simulink model "v01_aa_rii_16r4t11f_ver121sa"  (Simulink 7.6)
settings: Solver=ode45  StartTime=0.0  StopTime=128000  RelTol=1e-3
undefined symbols (not defined in the file or in its folder): sfp
libraries: xbsIndex_r4 x27287, monroe_library x154, xbsReplacements_r4 x78, casper_library_misc x33, simulink x32, dspsigops x32, xps_library x24
(part 24 of 178)

== system v01_aa_rii_16r4t11f_ver121sa/pfb_fir_real2/coeff_gen_6_9 ==
  sync : Inport
  Counter0 : xbsIndex_r4/Counter  cnt_type=Free Running  cnt_to=Inf  operation=Up  start_count=8  cnt_by_val=1  arith_type=Unsigned  n_bits=7  bin_pt=0  load_pin=off  rst=on  en=off  explicit_period=on  period=1  dbl_ovrd=off  use_behavioral_HDL=off  implementation=Fabric  block_type=counter
  Counter1 : xbsIndex_r4/Counter  cnt_type=Free Running  cnt_to=Inf  operation=Down  start_count=119  cnt_by_val=1  arith_type=Unsigned  n_bits=7  bin_pt=0  load_pin=off  rst=on  en=off  explicit_period=on  period=1  dbl_ovrd=off  use_behavioral_HDL=off  implementation=Fabric  block_type=counter
  Delay31 : xbsIndex_r4/Delay  rst=off  infoeditControl=Selection of Reset will increase slice count due to use of real FFs and instead of SRLs  en=off  latency=2  dbl_ovrd=off  reg_retiming=off  block_type=delay
  Delay32 : xbsIndex_r4/Delay  rst=off  infoeditControl=Selection of Reset will increase slice count due to use of real FFs and instead of SRLs  en=off  latency=1  dbl_ovrd=off  reg_retiming=off  block_type=delay
  Delay33 : xbsIndex_r4/Delay  rst=off  infoeditControl=Selection of Reset will increase slice count due to use of real FFs and instead of SRLs  en=off  latency=1  dbl_ovrd=off  reg_retiming=off  block_type=delay
  Delay34 : xbsIndex_r4/Delay  rst=off  infoeditControl=Selection of Reset will increase slice count due to use of real FFs and instead of SRLs  en=off  latency=2  dbl_ovrd=off  reg_retiming=off  block_type=delay
  coeff_ram_1 : xbsIndex_r4/Dual Port RAM  depth=128  initVector=[-0.000127160545753068 -0.000442154443954354 -0.000760500021931522 -0.00108286593169467 -0.00140992727613395 -0.00174236399262348 -0.00208085920782171 -0.00242609756580559 -0.00277876353172613 -0.0031395396732198 -0.00350910492185494 -0.00388813281693438 -0.00427728973401434 -0.00467723310053645 -0.00508860960100282 -0.00551205337415503 -0.00594818420464534 -0.00639760571171294 -0.00686090353739995 -0.00733864353685997 -0.00783136997332745 -0.00833960372032831 -0.00886384047372118 -0.00940454897616429 -0.00996216925660562 -0.0105371108873929 -0.0111297512615966 -0.0117404338931308 -0.012369466742247 -0.0130171205689619 -0.0136836273169615 -0.0143691785305073 -0.0150739238068405 -0.0157979692865596 -0.0165413761844115 -0.0173041593629059 -0.0180862859511236 -0.0188876740110524 -0.0197081912537385 -0.0205476538074967 -0.0214058250403737 -0.0222824144390066 -0.0231770765459626 -0.0240894099575895 -0.0250189563843456 -0.025965199775512 -0.0269275655101279 -0.0279054196559168 -0.0288980682979016 -0.0299047569383341 -0.0309246699694849 -0.0319569302207636 -0.0330005985815571 -0.0340546737010912 -0.0351180917665341 -0.0361897263604755 -0.0372683883988247 -0.0383528261500787 -0.0394417253368223 -0.0405337093202253 -0.0416273393682069 -0.042721115007841 -0.0438134744624772 -0.0449027951739552 -0.0459873944101843 -0.0470655299582668 -0.0481354009032336 -0.0491951484923634 -0.0502428570849517 -0.0512765551872898 -0.0522942165725149 -0.0532937614848831 -0.0542730579279157 -0.0552299230357647 -0.0561621245270369 -0.0570673822402172 -0.0579433697497217 -0.0587877160615143 -0.0595980073871155 -0.0603717889947299 -0.0611065671361221 -0.0617998110477661 -0.0624489550247014 -0.0630514005654251 -0.0636045185860608 -0.0641056517019449 -0.0645521165746833 -0.0649412063226375 -0.0652701929927136 -0.0655363300912374 -0.0657368551716176 -0.0658689924764135 -0.0659299556313471 -0.065916950388718 -0.0658271774176071 -0.065657835138181 -0.0654061225973403 -0.0650692423828866 -0.0646444035733198 -0.0641288247203163 -0.063519736860879 -0.0628143865560972 -0.0620100389534008 -0.0611039808691464 -0.0600935238883252 -0.0589760074781449 -0.0577488021121952 -0.0564093124018754 -0.0549549802317284 -0.0533832878953016 -0.0516917612281279 -0.0498779727344022 -0.0479395447039121 -0.0458741523157694 -0.0436795267254782 -0.0413534581318762 -0.0388937988204769 -0.0362984661797526 -0.0335654456868968 -0.0306927938596214 -0.0276786411705567 -0.0245211949208399 -0.021218742069504 -0.0177696520153009 -0.0141723793276274 -0.0104254664232543 -0.00652754618559848 -0.00247734452331941]  distributed_mem=Block RAM  init_a=0  init_b=0  rst_a=off  rst_b=off  en_a=off  en_b=off  latency=2  write_mode_A=No Read On Write  write_mode_B=No Read On Write  dbl_ovrd=off  optimize=Area  block_type=dpram
  coeff_ram_2 : xbsIndex_r4/Dual Port RAM  depth=128  initVector=[0.00172631713493355 0.00608451941274449 0.0105982426281969 0.0152683645091936 0.0200956579847837 0.0250807890553853 0.0302243147447241 0.0355266811362388 0.0409882214966272 0.0466091544891328 0.0523895824790868 0.0583294899341408 0.0644287419215347 0.0706870827046562 0.0771041344410556 0.0836793959839828 0.0904122417894169 0.0973019209304556 0.104347556220831 0.111548143449209 0.118902550725827 0.12640951794291 0.13406765635019 0.141875448246753 0.149831246790306 0.157933275924849 0.166179630427611 0.174568276076004 0.183097049935197 0.191763660766828 0.200565689559221 0.209500590179345 0.218565690146664 0.227758191528855 0.237075171959277 0.246513585775932 0.256070265281535 0.265741922124186 0.275525148798008 0.285416420262988 0.295412095683125 0.305508420281884 0.3157015273138 0.325987440150977 0.336362074483092 0.346821240629391 0.357360645961044 0.367975897432114 0.378662504217261 0.389415880454205 0.400231348088824 0.411104139820711 0.422029402146814 0.433002198500771 0.444017512485363 0.455070251195456 0.466155248628685 0.477267269181019 0.488401011224277 0.499551110762549 0.510712145164397 0.521878636967618 0.533045057753271 0.544205832085582 0.555355341514271 0.566487928635764 0.577597901209684 0.588679536326957 0.599727084625781 0.610734774551675 0.621696816657757 0.632607407941328 0.643460736212841 0.654250984493227 0.664972335435562 0.675618975766998 0.686185100746851 0.696664918636714 0.70705265517845 0.717342558075884 0.727528901476001 0.73760599044547 0.747568165438271 0.757409806750234 0.767125338956278 0.776709235326166 0.786156022214578 0.795460283421358 0.804616664517761 0.813619877134603 0.822464703208207 0.831145999180092 0.83965870014639 0.847997823952992 0.856158475232522 0.864135849379227 0.87192523645797 0.879522025043565 0.886921705986721 0.894119876102989 0.901112241781117 0.907894622507326 0.914462954302096 0.920813293066113 0.926941817832139 0.932844833919638 0.938518775989079 0.943960210992963 0.949165841020668 0.95413250603436 0.958857186493295 0.963337005863931 0.967569233013425 0.971551284484145 0.975280726646996 0.978755277731429 0.981972809730167 0.984931350176759 0.987629083794236 0.990064354013247 0.992235664358174 0.99414167969989 0.995781227373902 0.997153298162806 0.998257047142064 0.999091794388297 0.999657025549383 0.999952392275796]  distributed_mem=Block RAM  init_a=0  init_b=0  rst_a=off  rst_b=off  en_a=off  en_b=off  latency=2  write_mode_A=No Read On Write  write_mode_B=No Read On Write  dbl_ovrd=off  optimize=Area  block_type=dpram
  coeff_ram_3 : xbsIndex_r4/Dual Port RAM  depth=128  initVector=[0.99997771251278 0.999732970653049 0.999218317549884 0.998434070390593 0.997380712430478 0.996058892587554 0.994469424898436 0.992613287835907 0.990491623488855 0.988105736605385 0.985457093500027 0.982547320826139 0.97937820421469 0.975951686780769 0.972269867499286 0.968334999451451 0.964149487943763 0.959715888501334 0.955036904737536 0.950115386102039 0.944954325509455 0.939556856850904 0.933926252390927 0.928065920052311 0.921979400591453 0.915670364667029 0.909142609804831 0.902400057261718 0.895446748791748 0.888286843317619 0.880924613510665 0.873364442282729 0.865610819193305 0.857668336775436 0.84954168678393 0.841235656369505 0.832755124182575 0.824105056410419 0.815290502751561 0.806316592331227 0.797188529561808 0.787911589952287 0.778491115870662 0.768932512263395 0.759241242335996 0.749422823198839 0.73948282148236 0.729426848925803 0.719260557943686 0.708989637174192 0.698619807013667 0.688156815141452 0.677606432039239 0.666974446509149 0.656266661194729 0.645488888109049 0.634646944174036 0.623746646775204 0.61279380933588 0.601794236914998 0.590753721832511 0.579678039326436 0.568572943245462 0.557444161781071 0.546297393243007 0.535138301881911 0.523972513762873 0.512805612693577 0.501643136210658 0.490490571627834 0.47935335214926 0.468236853051529 0.457146387937616 0.446087205066007 0.435064483758156 0.424083330887324 0.413148777451761 0.402265775235104 0.391439193556746 0.380673816114852 0.369974337924576 0.359345362353949 0.348791398259757 0.338316857225683 0.327926050904795 0.317623188468427 0.307412374163318 0.297297604978796 0.287282768425655 0.277371640428268 0.267567883331338 0.257875044022575 0.248296552172474 0.238835718592215 0.22949573371062 0.22027966617093 0.211190461548078 0.202230941186993 0.193403801162323 0.184711611359875 0.176156814679917 0.167741726362362 0.159468533433743 0.151339294275747 0.143355938314956 0.135520265833324 0.127833947898791 0.120298526415308 0.112915414291441 0.105685895726591 0.0986111266137515 0.091692135057604 0.0849298220066585 0.0783249619980033 0.0718782040131405 0.065590072443267 0.059460968162254 0.0534911697054773 0.0476808345525469 0.0420300005118858 0.0365385872050146 0.0312063976483006 0.0260331199298443 0.0210183289790836 0.0161614884266173 0.0114619525516619 0.00691896831448336 0.00253167747106489]  distributed_mem=Block RAM  init_a=0  init_b=0  rst_a=off  rst_b=off  en_a=off  en_b=off  latency=2  write_mode_A=No Read On Write  write_mode_B=No Read On Write  dbl_ovrd=off  optimize=Area  block_type=dpram
  coeff_ram_4 : xbsIndex_r4/Dual Port RAM  depth=128  initVector=[-0.00170088123279474 -0.00577976979082941 -0.00970614859204155 -0.0134812740058263 -0.0171064958995449 -0.0205832550916594 -0.0239130807435938 -0.0270975876935306 -0.0301384737353973 -0.0330375168463324 -0.0357965723659607 -0.0384175701308345 -0.0409025115674262 -0.0432534667470823 -0.0454725714063674 -0.0475620239362406 -0.0495240823435232 -0.0513610611881183 -0.0530753284994518 -0.0546693026756019 -0.0561454493685798 -0.0575062783592176 -0.0587543404251082 -0.0598922242050244 -0.0609225530632278 -0.0618479819570521 -0.0626711943111221 -0.0633948989015359 -0.0640218267533063 -0.0645547280543195 -0.0649963690890256 -0.0653495291950351 -0.0656169977457445 -0.065801571162064 -0.0659060499562657 -0.0659332358109143 -0.0658859286957793 -0.0657669240255663 -0.0655790098612383 -0.0653249641576253 -0.0650075520599546 -0.0646295232518529 -0.0641936093572988 -0.0637025213989213 -0.0631589473149624 -0.0625655495371307 -0.061924962631494 -0.0612397910044664 -0.0605126066758539 -0.0597459471208361 -0.0589423131826611 -0.0581041670577407 -0.0572339303547331 -0.0563339822291054 -0.0554066575945648 -0.0544542454126514 -0.0534789870616819 -0.0524830747861326 -0.0514686502274456 -0.0504378030371417 -0.0493925695730179 -0.0483349316791022 -0.0472668155499383 -0.0461900906796629 -0.0451065688962393 -0.0440180034811031 -0.0429260883743734 -0.0418324574656808 -0.0407386839705573 -0.0396462798922348 -0.0385566955685944 -0.0374713193039103 -0.0363914770849303 -0.0353184323807401 -0.0342533860257561 -0.0331974761851017 -0.0321517784015221 -0.0311173057229034 -0.0300950089093698 -0.0290857767188417 -0.028090436269853 -0.0271097534803362 -0.0261444335810056 -0.0251951217018821 -0.0242624035304276 -0.0233468060396795 -0.0224487982847001 -0.0215687922655849 -0.0207071438552046 -0.0198641537897875 -0.019040068720387 -0.0182350823232145 -0.0174493364667647 -0.0166829224335973 -0.0159358821945947 -0.0152082097334586 -0.0144998524191667 -0.0138107124240637 -0.0131406481852233 -0.0124894759066787 -0.0118569711000848 -0.0112428701613468 -0.0106468719807189 -0.0100686395838558 -0.00950780180127588 -0.00896395496367837 -0.00843666462054276 -0.00792546727942595 -0.00742987216336619 -0.00694936298379714 -0.00648339972637473 -0.00603142044712065 -0.00559284307629214 -0.00516706722739558 -0.00475347600877312 -0.00435143783520638 -0.00396030823699884 -0.00357943166401998 -0.00320814328221772 -0.00284577076013319 -0.00249163604298165 -0.00214505711189663 -0.00180534972596959 -0.00147182914475696 -0.00114381182896693 -0.0008206171170831 -0.00050156887572838 -0.000185997121622189]  distributed_mem=Block RAM  init_a=0  init_b=0  rst_a=off  rst_b=off  en_a=off  en_b=off  latency=2  write_mode_A=No Read On Write  write_mode_B=No Read On Write  dbl_ovrd=off  optimize=Area  block_type=dpram
  const_din : xbsIndex_r4/Constant  const=0  gui_display_data_type=Fixed-point  arith_type=Signed (2's comp)  n_bits=18  bin_pt=17  preci_type=Single  exp_width=8  frac_width=24  explicit_period=on  period=1  equ=P=C  opselect=C  inp2=PCIN>>17  opr=+  inp1=P  carry=CIN  dbl_ovrd=off  block_type=constant
  const_we : xbsIndex_r4/Constant  const=0  gui_display_data_type=Fixed-point  arith_type=Boolean  n_bits=16  bin_pt=14  preci_type=Single  exp_width=8  frac_width=24  explicit_period=on  period=1  equ=P=C  opselect=C  inp2=PCIN>>17  opr=+  inp1=P  carry=CIN  dbl_ovrd=off  block_type=constant
  cram_lower : Subsystem
  cram_upper : Subsystem
  sync_out : Outport
  coeff : Outport  Port=2
  coeff_rev : Outport  Port=3
  wires:
  sync/1 -> Counter1/1, Counter0/1, sync_out/1
  Counter0/1 -> coeff_ram_4/1, coeff_ram_3/1, Delay32/1, Delay31/1
  const_din/1 -> coeff_ram_4/5, coeff_ram_4/2, coeff_ram_3/5, coeff_ram_3/2, coeff_ram_2/5, coeff_ram_2/2, coeff_ram_1/5, coeff_ram_1/2
  const_we/1 -> coeff_ram_4/6, coeff_ram_4/3, coeff_ram_3/6, coeff_ram_3/3, coeff_ram_2/6, coeff_ram_2/3, coeff_ram_1/6, coeff_ram_1/3
  Counter1/1 -> Delay34/1, Delay33/1, coeff_ram_2/4, coeff_ram_1/4
  cram_lower/1(out) -> coeff_rev/1
  cram_upper/1(out) -> coeff/1
  coeff_ram_1/2 -> cram_lower/4(in3)
  coeff_ram_2/2 -> cram_lower/3(in2)
  coeff_ram_3/2 -> cram_lower/2(in1)
  coeff_ram_4/2 -> cram_lower/1(in0)
  coeff_ram_4/1 -> cram_upper/4(in3)
  coeff_ram_3/1 -> cram_upper/3(in2)
  coeff_ram_2/1 -> cram_upper/2(in1)
  coeff_ram_1/1 -> cram_upper/1(in0)
  Delay34/1 -> coeff_ram_4/4
  Delay33/1 -> coeff_ram_3/4
  Delay32/1 -> coeff_ram_2/1
  Delay31/1 -> coeff_ram_1/1

== system v01_aa_rii_16r4t11f_ver121sa/pfb_fir_real2/coeff_gen_7_8 ==
  sync : Inport
  Counter0 : xbsIndex_r4/Counter  cnt_type=Free Running  cnt_to=Inf  operation=Up  start_count=8  cnt_by_val=1  arith_type=Unsigned  n_bits=7  bin_pt=0  load_pin=off  rst=on  en=off  explicit_period=on  period=1  dbl_ovrd=off  use_behavioral_HDL=off  implementation=Fabric  block_type=counter
  Counter1 : xbsIndex_r4/Counter  cnt_type=Free Running  cnt_to=Inf  operation=Down  start_count=119  cnt_by_val=1  arith_type=Unsigned  n_bits=7  bin_pt=0  load_pin=off  rst=on  en=off  explicit_period=on  period=1  dbl_ovrd=off  use_behavioral_HDL=off  implementation=Fabric  block_type=counter
  Delay36 : xbsIndex_r4/Delay  rst=off  infoeditControl=Selection of Reset will increase slice count due to use of real FFs and instead of SRLs  en=off  latency=2  dbl_ovrd=off  reg_retiming=off  block_type=delay
  Delay37 : xbsIndex_r4/Delay  rst=off  infoeditControl=Selection of Reset will increase slice count due to use of real FFs and instead of SRLs  en=off  latency=1  dbl_ovrd=off  reg_retiming=off  block_type=delay
  Delay38 : xbsIndex_r4/Delay  rst=off  infoeditControl=Selection of Reset will increase slice count due to use of real FFs and instead of SRLs  en=off  latency=1  dbl_ovrd=off  reg_retiming=off  block_type=delay
  Delay39 : xbsIndex_r4/Delay  rst=off  infoeditControl=Selection of Reset will increase slice count due to use of real FFs and instead of SRLs  en=off  latency=2  dbl_ovrd=off  reg_retiming=off  block_type=delay
  coeff_ram_1 : xbsIndex_r4/Dual Port RAM  depth=128  initVector=[-0.000146762015096983 -0.000461945948052194 -0.000780523132382771 -0.00110316267006006 -0.00143054001379427 -0.00176333534877651 -0.00210223194574943 -0.00244791448754683 -0.00280106737129305 -0.00316237298849928 -0.00353250998533891 -0.00391215150542528 -0.00430196341745465 -0.00470260253011307 -0.00511471479667937 -0.00553893351178669 -0.00597587750283262 -0.00642614931855231 -0.00689033341729023 -0.00736899435752464 -0.00786267499321385 -0.0083718946765453 -0.00889714747067734 -0.00943890037506898 -0.00999759156599516 -0.0105736286548443 -0.0111673869667904 -0.0117792078424244 -0.0124093969649192 -0.0130582227152872 -0.0137259145582748 -0.0144126614614124 -0.0151186103497212 -0.0158438645985451 -0.0165884825669475 -0.0173524761740819 -0.0181358095209027 -0.0189383975595475 -0.0197601048126773 -0.0206007441450138 -0.0214600755892652 -0.0223378052285791 -0.0232335841376052 -0.0241470073841942 -0.0250776130936957 -0.0260248815777572 -0.0269882345294571 -0.0279670342865377 -0.0289605831644304 -0.0299681228606948 -0.0309888339324118 -0.0320218353479964 -0.0330661841148128 -0.0341208749838907 -0.0351848402329585 -0.036256949528918 -0.0373360098708014 -0.0384207656141553 -0.0395098985777058 -0.0406020282330669 -0.0416957119781535 -0.0427894454948693 -0.0438816631915359 -0.044970738730435 -0.0460549856407311 -0.0471326580169441 -0.0482019513030358 -0.0492610031620756 -0.0503078944313416 -0.0513406501626167 -0.0523572407473282 -0.0533555831260807 -0.0543335420820237 -0.0552889316173935 -0.056219516412465 -0.057123013366042 -0.0579970932165166 -0.0588393822424205 -0.0596474640412915 -0.060418881385577 -0.061151138154196 -0.0618417013382798 -0.0624880031195173 -0.0630874430194303 -0.0636373901178102 -0.0641351853384555 -0.0645781438002524 -0.0649635572315565 -0.0652886964457401 -0.0655508138756844 -0.0657471461649141 -0.0658749168129847 -0.065931338872657 -0.0659136176963139 -0.0658189537289998 -0.0656445453453909 -0.0653875917279336 -0.0650452957833243 -0.0646148670944361 -0.06409352490474 -0.0634785011322087 -0.0627670434096362 -0.0619564181482566 -0.0610439136214937 -0.0600268430656328 -0.05890254779416 -0.0576684003224797 -0.0563218074996844 -0.0548602136440219 -0.0532811036786751 -0.0515820062644503 -0.0497604969259452 -0.0478142011677561 -0.0457407975772691 -0.0435380209105723 -0.0412036651580236 -0.0387355865860033 -0.0361317067513902 -0.0333900154853026 -0.0305085738426582 -0.0274855170141237 -0.0243190571970391 -0.0210074864219326 -0.0175491793312596 -0.0139425959070377 -0.0101862841440803 -0.00627888266556972 -0.00221912327775564]  distributed_mem=Block RAM  init_a=0  init_b=0  rst_a=off  rst_b=off  en_a=off  en_b=off  latency=2  write_mode_A=No Read On Write  write_mode_B=No Read On Write  dbl_ovrd=off  optimize=Area  block_type=dpram
  coeff_ram_2 : xbsIndex_r4/Dual Port RAM  depth=128  initVector=[0.00199416653939185 0.00636206120869445 0.0108855347025095 0.0155654582627141 0.0204025981968801 0.0253976137535212 0.0305510550792318 0.0358633612604601 0.0413348584525883 0.0469657580989122 0.0527561552420312 0.0587060269300772 0.0648152307201241 0.0710835032810244 0.0775104590978362 0.0840955892798959 0.0908382604745039 0.0977377138880848 0.104793064416578 0.112003299886717 0.119367280409732 0.126883737848928 0.134551275402435 0.142368367302369 0.150333358631466 0.158444465258183 0.166699773891124 0.175097242253503 0.183634699378287 0.192309846024486 0.201120255214974 0.210063372896065 0.21913651871898 0.228336886943181 0.237661547461441 0.247107446946385 0.256671410118117 0.266350141132408 0.276140225088811 0.286038129657917 0.296040206826872 0.306142694762123 0.316341719788239 0.32663329848155 0.337013339877193 0.347477647788056 0.358021923233973 0.36864176697942 0.379332682177829 0.390090077120521 0.400909268088159 0.411785482302493 0.422713860976064 0.433689462457427 0.444707265469343 0.455762172437285 0.466849012905507 0.477962547037825 0.489097469200151 0.500248411621744 0.511409948132039 0.522576597969841 0.533742829661563 0.544903064965141 0.556051682876141 0.567183023692534 0.578291393134523 0.589371066515746 0.600416292962115 0.611421299674493 0.622380296231356 0.633287478927515 0.644137035144977 0.65492314775191 0.665639999525697 0.676281777595997 0.686842677903704 0.697316909671679 0.707698699883091 0.717982297763205 0.728161979260409 0.738232051522307 0.748186857362649 0.758020779714911 0.767728246068318 0.777303732882114 0.786741769973893 0.79603694487784 0.805183907168721 0.814177372747513 0.823012128084576 0.831683034416324 0.840185031891353 0.848513143662084 0.856662479917952 0.864628241856301 0.872405725587129 0.879990325967942 0.887377540364997 0.894562972337311 0.901542335239865 0.90831145574251 0.914866277261172 0.921202863298015 0.927317400687328 0.933206202743959 0.938865712311265 0.94429250470557 0.949483290554292 0.954434918524957 0.959144377942428 0.963608801291808 0.967825466604565 0.971791799725535 0.975505376458598 0.978963924588919 0.982165325779773 0.985107617342099 0.98778899387504 0.99020780877587 0.992362575617817 0.994251969394439 0.995874827629316 0.997230151349991 0.99831710592518 0.999135021764442 0.999683394879622 0.999961887307505]  distributed_mem=Block RAM  init_a=0  init_b=0  rst_a=off  rst_b=off  en_a=off  en_b=off  latency=2  write_mode_A=No Read On Write  write_mode_B=No Read On Write  dbl_ovrd=off  optimize=Area  block_type=dpram
  coeff_ram_3 : xbsIndex_r4/Dual Port RAM  depth=128  initVector=[0.999970327393274 0.999708709934495 0.999177196185482 0.998376113722032 0.997305956166682 0.995967382774741 0.994361217881507 0.992488450211219 0.990350232048417 0.987947878272525 0.985282865256609 0.982356829631386 0.979171566915702 0.97572903001482 0.972031327587999 0.968080722286951 0.96387962886692 0.959430612172221 0.954736384998219 0.949799805831843 0.944623876472843 0.939211739538114 0.933566675851536 0.927692101721869 0.921591566111373 0.915268747697902 0.908727451833345 0.90197160740138 0.895005263577584 0.887832586495072 0.880457855818883 0.872885461232459 0.865119898839605 0.857165767485426 0.849027764999809 0.840710684367061 0.832219409825417 0.823558912900179 0.814734248374296 0.805750550200266 0.796613027357295 0.787326959657669 0.77789769350637 0.768330637617979 0.758631258694962 0.748805077071453 0.738857662326676 0.728794628872177 0.718621631517039 0.708344361015273 0.697968539599602 0.687499916505816 0.676944263491929 0.666307370356321 0.655595040459053 0.644813086250539 0.633967324811734 0.623063573409952 0.61210764507445 0.601105344195828 0.590062462153306 0.578984772973868 0.567878029027235 0.556747956760575 0.545600252476811 0.534440578160332 0.523274557353848 0.512107771090063 0.500945753881801 0.489793989774102 0.478657908461773 0.467542881475792 0.456454218441849 0.445397163414288 0.434376891288555 0.423398504295229 0.412467028578566 0.401587410862443 0.390764515206442 0.380003119854737 0.369307914180353 0.358683495727226 0.348134367352417 0.337664934470704 0.327279502403677 0.316982273835315 0.306777346375963 0.296668710236444 0.286660246013975 0.276755722591397 0.266958795151142 0.257273003305192 0.247701769342212 0.238248396592867 0.228916067914241 0.219707844294133 0.210626663575877 0.201675339304225 0.192856559692668 0.184172886712494 0.175626755303703 0.167220472707813 0.158956217922433 0.150836041277392 0.142861864132034 0.135035478693229 0.127358547953464 0.119832605748316 0.112459056932427 0.105239177673046 0.0981741158600261 0.0912648916310948 0.084512398011068 0.0779174016635925 0.07148054375387 0.0652023409207219 0.0590831863562395 0.0531233509911659 0.0473229847840524 0.041682118112133 0.0362006632617669 0.0308784160162031 0.0257150573383326 0.0207101551460066 0.0158631661774126 0.011173437943922 0.00664021076774132 0.0022626199016281]  distributed_mem=Block RAM  init_a=0  init_b=0  rst_a=off  rst_b=off  en_a=off  en_b=off  latency=2  write_mode_A=No Read On Write  write_mode_B=No Read On Write  dbl_ovrd=off  optimize=Area  block_type=dpram
  coeff_ram_4 : xbsIndex_r4/Dual Port RAM  depth=128  initVector=[-0.00196030227214131 -0.00602962396643271 -0.00994651164048777 -0.0137122275807361 -0.0173281274142497 -0.0207956575579549 -0.0241163526067695 -0.0272918326638788 -0.030323800616404 -0.0332140393597596 -0.0359644089740277 -0.0385768438557084 -0.0410533498082359 -0.0433960010946682 -0.0456069374559821 -0.0476883610984166 -0.0496425336533236 -0.051471773112987 -0.0531784507458805 -0.0547649879948297 -0.0562338533615425 -0.0575875592809619 -0.0588286589888855 -0.0599597433862781 -0.0609834379036862 -0.0619023993691383 -0.0627193128828887 -0.063436888702333 -0.0640578591403863 -0.0645849754805813 -0.0650210049120985 -0.0653687274878981 -0.065630933109075 -0.0658104185385061 -0.0659099844468056 -0.0659324324935443 -0.0658805624466314 -0.0657571693426894 -0.0655650406911897 -0.065306953725044 -0.0649856727002773 -0.0646039462473303 -0.0641645047764638 -0.0636700579396572 -0.0631232921513118 -0.062526868169983 -0.0618834187432817 -0.0611955463179954 -0.0604658208173894 -0.0596967774875561 -0.0588909148145869 -0.0580506925142456 -0.0571785295957271 -0.0562768025009831 -0.0553478433210022 -0.0543939380903286 -0.0534173251610023 -0.0524201936570026 -0.0514046820101748 -0.0503728765785135 -0.0493268103475761 -0.0482684617156931 -0.0471997533635396 -0.0461225512085252 -0.0450386634443597 -0.0439498396660427 -0.0428577700804262 -0.0417640848023921 -0.0406703532365864 -0.0395780835445478 -0.0384887221969679 -0.0374036536107213 -0.0363241998701992 -0.0352516205323896 -0.0341871125150421 -0.0331318100671657 -0.0320867848210099 -0.0310530459245885 -0.0300315402537142 -0.0290231527024214 -0.0280287065505702 -0.0270489639073352 -0.0260846262292031 -0.0251363349110189 -0.0242046719485435 -0.0232901606709079 -0.0223932665412744 -0.0215143980239454 -0.0206539075160887 -0.0198120923421836 -0.0189891958092265 -0.0181854083206757 -0.0174008685470547 -0.0166356646510793 -0.0158898355651202 -0.0151633723187648 -0.0144562194141947 -0.0137682762470507 -0.0130993985704197 -0.0124493999995389 -0.0118180535547789 -0.0112050932404367 -0.010610215656844 -0.0100330816432682 -0.00947331794906695 -0.00893051893053586 -0.00840424827087738 -0.00789404072070559 -0.00739940385649554 -0.00691981985438059 -0.00645474727669994 -0.00600362286870091 -0.0055658633628058 -0.00514086728786142 -0.00472801678080149 -0.00432667939816694 -0.00393620992494696 -0.00355595217822514 -0.003185240803139 -0.00282340305868871 -0.00246976059096078 -0.00212363119136582 -0.00178433053752527 -0.00145117391448125 -0.00112347791394463 -0.000800562109341241 -0.000481750704462836 -0.000166374153578663]  distributed_mem=Block RAM  init_a=0  init_b=0  rst_a=off  rst_b=off  en_a=off  en_b=off  latency=2  write_mode_A=No Read On Write  write_mode_B=No Read On Write  dbl_ovrd=off  optimize=Area  block_type=dpram
  const_din : xbsIndex_r4/Constant  const=0  gui_display_data_type=Fixed-point  arith_type=Signed (2's comp)  n_bits=18  bin_pt=17  preci_type=Single  exp_width=8  frac_width=24  explicit_period=on  period=1  equ=P=C  opselect=C  inp2=PCIN>>17  opr=+  inp1=P  carry=CIN  dbl_ovrd=off  block_type=constant
  const_we : xbsIndex_r4/Constant  const=0  gui_display_data_type=Fixed-point  arith_type=Boolean  n_bits=16  bin_pt=14  preci_type=Single  exp_width=8  frac_width=24  explicit_period=on  period=1  equ=P=C  opselect=C  inp2=PCIN>>17  opr=+  inp1=P  carry=CIN  dbl_ovrd=off  block_type=constant
  cram_lower : Subsystem
  cram_upper : Subsystem
  sync_out : Outport
  coeff : Outport  Port=2
  coeff_rev : Outport  Port=3
  wires:
  sync/1 -> Counter1/1, Counter0/1, sync_out/1
  Counter0/1 -> coeff_ram_4/1, coeff_ram_3/1, Delay37/1, Delay36/1
  const_din/1 -> coeff_ram_4/5, coeff_ram_4/2, coeff_ram_3/5, coeff_ram_3/2, coeff_ram_2/5, coeff_ram_2/2, coeff_ram_1/5, coeff_ram_1/2
  const_we/1 -> coeff_ram_4/6, coeff_ram_4/3, coeff_ram_3/6, coeff_ram_3/3, coeff_ram_2/6, coeff_ram_2/3, coeff_ram_1/6, coeff_ram_1/3
  Counter1/1 -> Delay39/1, Delay38/1, coeff_ram_2/4, coeff_ram_1/4
  cram_lower/1(out) -> coeff_rev/1
  cram_upper/1(out) -> coeff/1
  coeff_ram_1/2 -> cram_lower/4(in3)
  coeff_ram_2/2 -> cram_lower/3(in2)
  coeff_ram_3/2 -> cram_lower/2(in1)
  coeff_ram_4/2 -> cram_lower/1(in0)
  coeff_ram_4/1 -> cram_upper/4(in3)
  coeff_ram_3/1 -> cram_upper/3(in2)
  coeff_ram_2/1 -> cram_upper/2(in1)
  coeff_ram_1/1 -> cram_upper/1(in0)
  Delay39/1 -> coeff_ram_4/4
  Delay38/1 -> coeff_ram_3/4
  Delay37/1 -> coeff_ram_2/1
  Delay36/1 -> coeff_ram_1/1

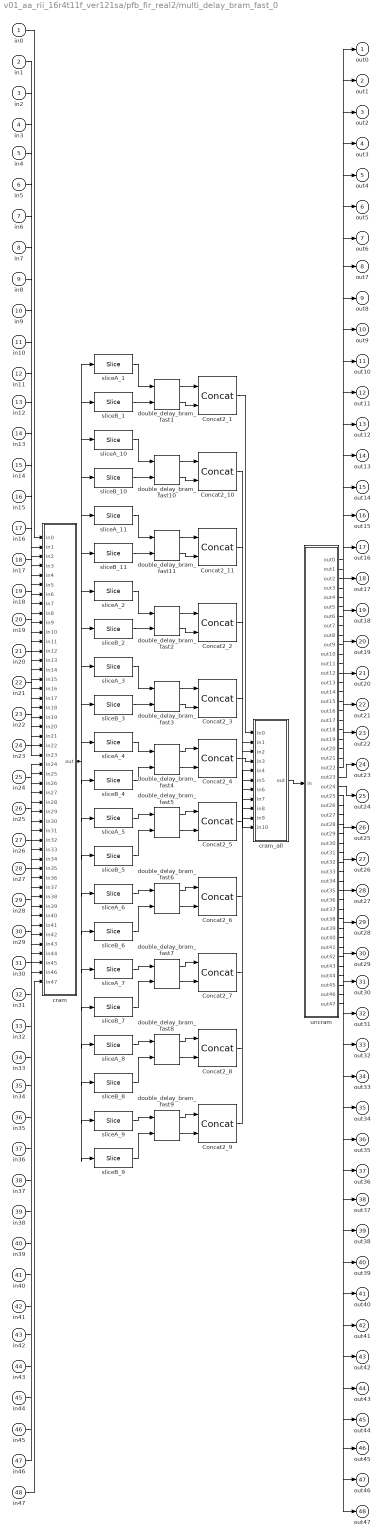
== system v01_aa_rii_16r4t11f_ver121sa/pfb_fir_real2/multi_delay_bram_fast_0 ==
  in0 : Inport
  in1 : Inport  Port=2
  in2 : Inport  Port=3
  in3 : Inport  Port=4
  in4 : Inport  Port=5
  in5 : Inport  Port=6
  in6 : Inport  Port=7
  in7 : Inport  Port=8
  in8 : Inport  Port=9
  in9 : Inport  Port=10
  in10 : Inport  Port=11
  in11 : Inport  Port=12
  in12 : Inport  Port=13
  in13 : Inport  Port=14
  in14 : Inport  Port=15
  in15 : Inport  Port=16
  in16 : Inport  Port=17
  in17 : Inport  Port=18
  in18 : Inport  Port=19
  in19 : Inport  Port=20
  in20 : Inport  Port=21
  in21 : Inport  Port=22
  in22 : Inport  Port=23
  in23 : Inport  Port=24
  in24 : Inport  Port=25
  in25 : Inport  Port=26
  in26 : Inport  Port=27
  in27 : Inport  Port=28
  in28 : Inport  Port=29
  in29 : Inport  Port=30
  in30 : Inport  Port=31
  in31 : Inport  Port=32
  in32 : Inport  Port=33
  in33 : Inport  Port=34
  in34 : Inport  Port=35
  in35 : Inport  Port=36
  in36 : Inport  Port=37
  in37 : Inport  Port=38
  in38 : Inport  Port=39
  in39 : Inport  Port=40
  in40 : Inport  Port=41
  in41 : Inport  Port=42
  in42 : Inport  Port=43
  in43 : Inport  Port=44
  in44 : Inport  Port=45
  in45 : Inport  Port=46
  in46 : Inport  Port=47
  in47 : Inport  Port=48
  Concat2_{1..11} (11 alike) : xbsIndex_r4/Concat  num_inputs=2  dbl_ovrd=off  block_type=concat
  cram : Subsystem
  cram_all : Subsystem
  double_delay_bram_fast{1..11} (11 alike) : monroe_library/double_delay_bram_fast  SystemSampleTime=-1  delay_len=128  bram_latency=2  register_counter=1
  sliceA_1 : xbsIndex_r4/Slice  nbits=18  boolean_output=off  mode=Upper Bit Location + Width  bit1=-0  base1=MSB of Input  bit0=-0  base0=MSB of Input  dbl_ovrd=off  block_type=slice
  sliceA_10 : xbsIndex_r4/Slice  nbits=18  boolean_output=off  mode=Upper Bit Location + Width  bit1=-324  base1=MSB of Input  bit0=-324  base0=MSB of Input  dbl_ovrd=off  block_type=slice
  sliceA_11 : xbsIndex_r4/Slice  nbits=12  boolean_output=off  mode=Lower Bit Location + Width  bit1=12  base1=LSB of Input  bit0=12  base0=LSB of Input  dbl_ovrd=off  block_type=slice
  sliceA_2 : xbsIndex_r4/Slice  nbits=18  boolean_output=off  mode=Upper Bit Location + Width  bit1=-36  base1=MSB of Input  bit0=-36  base0=MSB of Input  dbl_ovrd=off  block_type=slice
  sliceA_3 : xbsIndex_r4/Slice  nbits=18  boolean_output=off  mode=Upper Bit Location + Width  bit1=-72  base1=MSB of Input  bit0=-72  base0=MSB of Input  dbl_ovrd=off  block_type=slice
  sliceA_4 : xbsIndex_r4/Slice  nbits=18  boolean_output=off  mode=Upper Bit Location + Width  bit1=-108  base1=MSB of Input  bit0=-108  base0=MSB of Input  dbl_ovrd=off  block_type=slice
  sliceA_5 : xbsIndex_r4/Slice  nbits=18  boolean_output=off  mode=Upper Bit Location + Width  bit1=-144  base1=MSB of Input  bit0=-144  base0=MSB of Input  dbl_ovrd=off  block_type=slice
  sliceA_6 : xbsIndex_r4/Slice  nbits=18  boolean_output=off  mode=Upper Bit Location + Width  bit1=-180  base1=MSB of Input  bit0=-180  base0=MSB of Input  dbl_ovrd=off  block_type=slice
  sliceA_7 : xbsIndex_r4/Slice  nbits=18  boolean_output=off  mode=Upper Bit Location + Width  bit1=-216  base1=MSB of Input  bit0=-216  base0=MSB of Input  dbl_ovrd=off  block_type=slice
  sliceA_8 : xbsIndex_r4/Slice  nbits=18  boolean_output=off  mode=Upper Bit Location + Width  bit1=-252  base1=MSB of Input  bit0=-252  base0=MSB of Input  dbl_ovrd=off  block_type=slice
  sliceA_9 : xbsIndex_r4/Slice  nbits=18  boolean_output=off  mode=Upper Bit Location + Width  bit1=-288  base1=MSB of Input  bit0=-288  base0=MSB of Input  dbl_ovrd=off  block_type=slice
  sliceB_1 : xbsIndex_r4/Slice  nbits=18  boolean_output=off  mode=Upper Bit Location + Width  bit1=-18  base1=MSB of Input  bit0=-18  base0=MSB of Input  dbl_ovrd=off  block_type=slice
  sliceB_10 : xbsIndex_r4/Slice  nbits=18  boolean_output=off  mode=Upper Bit Location + Width  bit1=-342  base1=MSB of Input  bit0=-342  base0=MSB of Input  dbl_ovrd=off  block_type=slice
  sliceB_11 : xbsIndex_r4/Slice  nbits=12  boolean_output=off  mode=Lower Bit Location + Width  bit1=0  base1=LSB of Input  bit0=0  base0=LSB of Input  dbl_ovrd=off  block_type=slice
  sliceB_2 : xbsIndex_r4/Slice  nbits=18  boolean_output=off  mode=Upper Bit Location + Width  bit1=-54  base1=MSB of Input  bit0=-54  base0=MSB of Input  dbl_ovrd=off  block_type=slice
  sliceB_3 : xbsIndex_r4/Slice  nbits=18  boolean_output=off  mode=Upper Bit Location + Width  bit1=-90  base1=MSB of Input  bit0=-90  base0=MSB of Input  dbl_ovrd=off  block_type=slice
  sliceB_4 : xbsIndex_r4/Slice  nbits=18  boolean_output=off  mode=Upper Bit Location + Width  bit1=-126  base1=MSB of Input  bit0=-126  base0=MSB of Input  dbl_ovrd=off  block_type=slice
  sliceB_5 : xbsIndex_r4/Slice  nbits=18  boolean_output=off  mode=Upper Bit Location + Width  bit1=-162  base1=MSB of Input  bit0=-162  base0=MSB of Input  dbl_ovrd=off  block_type=slice
  sliceB_6 : xbsIndex_r4/Slice  nbits=18  boolean_output=off  mode=Upper Bit Location + Width  bit1=-198  base1=MSB of Input  bit0=-198  base0=MSB of Input  dbl_ovrd=off  block_type=slice
  sliceB_7 : xbsIndex_r4/Slice  nbits=18  boolean_output=off  mode=Upper Bit Location + Width  bit1=-234  base1=MSB of Input  bit0=-234  base0=MSB of Input  dbl_ovrd=off  block_type=slice
  sliceB_8 : xbsIndex_r4/Slice  nbits=18  boolean_output=off  mode=Upper Bit Location + Width  bit1=-270  base1=MSB of Input  bit0=-270  base0=MSB of Input  dbl_ovrd=off  block_type=slice
  sliceB_9 : xbsIndex_r4/Slice  nbits=18  boolean_output=off  mode=Upper Bit Location + Width  bit1=-306  base1=MSB of Input  bit0=-306  base0=MSB of Input  dbl_ovrd=off  block_type=slice
  uncram : Subsystem
  out0 : Outport
  out1 : Outport  Port=2
  out2 : Outport  Port=3
  out3 : Outport  Port=4
  out4 : Outport  Port=5
  out5 : Outport  Port=6
  out6 : Outport  Port=7
  out7 : Outport  Port=8
  out8 : Outport  Port=9
  out9 : Outport  Port=10
  out10 : Outport  Port=11
  out11 : Outport  Port=12
  out12 : Outport  Port=13
  out13 : Outport  Port=14
  out14 : Outport  Port=15
  out15 : Outport  Port=16
  out16 : Outport  Port=17
  out17 : Outport  Port=18
  out18 : Outport  Port=19
  out19 : Outport  Port=20
  out20 : Outport  Port=21
  out21 : Outport  Port=22
  out22 : Outport  Port=23
  out23 : Outport  Port=24
  out24 : Outport  Port=25
  out25 : Outport  Port=26
  out26 : Outport  Port=27
  out27 : Outport  Port=28
  out28 : Outport  Port=29
  out29 : Outport  Port=30
  out30 : Outport  Port=31
  out31 : Outport  Port=32
  out32 : Outport  Port=33
  out33 : Outport  Port=34
  out34 : Outport  Port=35
  out35 : Outport  Port=36
  out36 : Outport  Port=37
  out37 : Outport  Port=38
  out38 : Outport  Port=39
  out39 : Outport  Port=40
  out40 : Outport  Port=41
  out41 : Outport  Port=42
  out42 : Outport  Port=43
  out43 : Outport  Port=44
  out44 : Outport  Port=45
  out45 : Outport  Port=46
  out46 : Outport  Port=47
  out47 : Outport  Port=48
  wires:
  cram/1(out) -> sliceB_11/1, sliceA_11/1, sliceB_10/1, sliceA_10/1, sliceB_9/1, sliceA_9/1, sliceB_8/1, sliceA_8/1, sliceB_7/1, sliceA_7/1, sliceB_6/1, sliceA_6/1, sliceB_5/1, sliceA_5/1, sliceB_4/1, sliceA_4/1, sliceB_3/1, sliceA_3/1, sliceB_2/1, sliceA_2/1, sliceB_1/1, sliceA_1/1
  uncram/48(out47) -> out47/1
  uncram/47(out46) -> out46/1
  uncram/46(out45) -> out45/1
  uncram/45(out44) -> out44/1
  uncram/44(out43) -> out43/1
  uncram/43(out42) -> out42/1
  uncram/42(out41) -> out41/1
  uncram/41(out40) -> out40/1
  uncram/40(out39) -> out39/1
  uncram/39(out38) -> out38/1
  uncram/38(out37) -> out37/1
  uncram/37(out36) -> out36/1
  uncram/36(out35) -> out35/1
  uncram/35(out34) -> out34/1
  uncram/34(out33) -> out33/1
  uncram/33(out32) -> out32/1
  uncram/32(out31) -> out31/1
  uncram/31(out30) -> out30/1
  uncram/30(out29) -> out29/1
  uncram/29(out28) -> out28/1
  uncram/28(out27) -> out27/1
  uncram/27(out26) -> out26/1
  uncram/26(out25) -> out25/1
  uncram/25(out24) -> out24/1
  uncram/24(out23) -> out23/1
  uncram/23(out22) -> out22/1
  uncram/22(out21) -> out21/1
  uncram/21(out20) -> out20/1
  uncram/20(out19) -> out19/1
  uncram/19(out18) -> out18/1
  uncram/18(out17) -> out17/1
  uncram/17(out16) -> out16/1
  uncram/16(out15) -> out15/1
  uncram/15(out14) -> out14/1
  uncram/14(out13) -> out13/1
  uncram/13(out12) -> out12/1
  uncram/12(out11) -> out11/1
  uncram/11(out10) -> out10/1
  uncram/10(out9) -> out9/1
  uncram/9(out8) -> out8/1
  uncram/8(out7) -> out7/1
  uncram/7(out6) -> out6/1
  uncram/6(out5) -> out5/1
  uncram/5(out4) -> out4/1
  uncram/4(out3) -> out3/1
  uncram/3(out2) -> out2/1
  uncram/2(out1) -> out1/1
  uncram/1(out0) -> out0/1
  cram_all/1(out) -> uncram/1(in)
  Concat2_11/1 -> cram_all/11(in10)
  Concat2_10/1 -> cram_all/10(in9)
  Concat2_9/1 -> cram_all/9(in8)
  Concat2_8/1 -> cram_all/8(in7)
  Concat2_7/1 -> cram_all/7(in6)
  Concat2_6/1 -> cram_all/6(in5)
  Concat2_5/1 -> cram_all/5(in4)
  Concat2_4/1 -> cram_all/4(in3)
  Concat2_3/1 -> cram_all/3(in2)
  Concat2_2/1 -> cram_all/2(in1)
  Concat2_1/1 -> cram_all/1(in0)
  double_delay_bram_fast11/2 -> Concat2_11/2
  double_delay_bram_fast11/1 -> Concat2_11/1
  sliceB_11/1 -> double_delay_bram_fast11/2
  sliceA_11/1 -> double_delay_bram_fast11/1
  double_delay_bram_fast10/2 -> Concat2_10/2
  double_delay_bram_fast10/1 -> Concat2_10/1
  sliceB_10/1 -> double_delay_bram_fast10/2
  sliceA_10/1 -> double_delay_bram_fast10/1
  double_delay_bram_fast9/2 -> Concat2_9/2
  double_delay_bram_fast9/1 -> Concat2_9/1
  sliceB_9/1 -> double_delay_bram_fast9/2
  sliceA_9/1 -> double_delay_bram_fast9/1
  double_delay_bram_fast8/2 -> Concat2_8/2
  double_delay_bram_fast8/1 -> Concat2_8/1
  sliceB_8/1 -> double_delay_bram_fast8/2
  sliceA_8/1 -> double_delay_bram_fast8/1
  double_delay_bram_fast7/2 -> Concat2_7/2
  double_delay_bram_fast7/1 -> Concat2_7/1
  sliceB_7/1 -> double_delay_bram_fast7/2
  sliceA_7/1 -> double_delay_bram_fast7/1
  double_delay_bram_fast6/2 -> Concat2_6/2
  double_delay_bram_fast6/1 -> Concat2_6/1
  sliceB_6/1 -> double_delay_bram_fast6/2
  sliceA_6/1 -> double_delay_bram_fast6/1
  double_delay_bram_fast5/2 -> Concat2_5/2
  double_delay_bram_fast5/1 -> Concat2_5/1
  sliceB_5/1 -> double_delay_bram_fast5/2
  sliceA_5/1 -> double_delay_bram_fast5/1
  double_delay_bram_fast4/2 -> Concat2_4/2
  double_delay_bram_fast4/1 -> Concat2_4/1
  sliceB_4/1 -> double_delay_bram_fast4/2
  sliceA_4/1 -> double_delay_bram_fast4/1
  double_delay_bram_fast3/2 -> Concat2_3/2
  double_delay_bram_fast3/1 -> Concat2_3/1
  sliceB_3/1 -> double_delay_bram_fast3/2
  sliceA_3/1 -> double_delay_bram_fast3/1
  double_delay_bram_fast2/2 -> Concat2_2/2
  double_delay_bram_fast2/1 -> Concat2_2/1
  sliceB_2/1 -> double_delay_bram_fast2/2
  sliceA_2/1 -> double_delay_bram_fast2/1
  double_delay_bram_fast1/2 -> Concat2_1/2
  double_delay_bram_fast1/1 -> Concat2_1/1
  sliceB_1/1 -> double_delay_bram_fast1/2
  sliceA_1/1 -> double_delay_bram_fast1/1
  in47/1 -> cram/48(in47)
  in46/1 -> cram/47(in46)
  in45/1 -> cram/46(in45)
  in44/1 -> cram/45(in44)
  in43/1 -> cram/44(in43)
  in42/1 -> cram/43(in42)
  in41/1 -> cram/42(in41)
  in40/1 -> cram/41(in40)
  in39/1 -> cram/40(in39)
  in38/1 -> cram/39(in38)
  in37/1 -> cram/38(in37)
  in36/1 -> cram/37(in36)
  in35/1 -> cram/36(in35)
  in34/1 -> cram/35(in34)
  in33/1 -> cram/34(in33)
  in32/1 -> cram/33(in32)
  in31/1 -> cram/32(in31)
  in30/1 -> cram/31(in30)
  in29/1 -> cram/30(in29)
  in28/1 -> cram/29(in28)
  in27/1 -> cram/28(in27)
  in26/1 -> cram/27(in26)
  in25/1 -> cram/26(in25)
  in24/1 -> cram/25(in24)
  in23/1 -> cram/24(in23)
  in22/1 -> cram/23(in22)
  in21/1 -> cram/22(in21)
  in20/1 -> cram/21(in20)
  in19/1 -> cram/20(in19)
  in18/1 -> cram/19(in18)
  in17/1 -> cram/18(in17)
  in16/1 -> cram/17(in16)
  in15/1 -> cram/16(in15)
  in14/1 -> cram/15(in14)
  in13/1 -> cram/14(in13)
  in12/1 -> cram/13(in12)
  in11/1 -> cram/12(in11)
  in10/1 -> cram/11(in10)
  in9/1 -> cram/10(in9)
  in8/1 -> cram/9(in8)
  in7/1 -> cram/8(in7)
  in6/1 -> cram/7(in6)
  in5/1 -> cram/6(in5)
  in4/1 -> cram/5(in4)
  in3/1 -> cram/4(in3)
  in2/1 -> cram/3(in2)
  in1/1 -> cram/2(in1)
  in0/1 -> cram/1(in0)

== system v01_aa_rii_16r4t11f_ver121sa/rshpout/add_gen ==
  note: To storage
  note: prevent one extra
      | write to address 0
  note: Do one
      | capture by
      | default
  note: Count of bytes in BRAM
      | as well as done signal
  note: A total count of
      | bytes written to
      | BRAM since trig
  note: Force MSbit of count
      | to be '1' by negating busy
      | as counter bit may be '0' or '1'
  vin : Inport
  din : Inport  Port=2
  we : Inport  Port=3
  go : Inport  Port=4
  cont : Inport  Port=5
  init : Inport  Port=6
  Concat : xbsIndex_r4/Concat  num_inputs=3  dbl_ovrd=off  block_type=concat
      settings: block_version=10.1.3
  Convert : xbsIndex_r4/Convert  gui_display_data_type=Fixed-point  arith_type=Unsigned  n_bits=32-nsamples-log2(increment)-1  bin_pt=0  float_type=Single  exp_bits=8  fraction_bits=24  quantization=Truncate  overflow=Wrap  en=off  latency=0  dbl_ovrd=off  pipeline=off  block_type=convert
      settings: block_version=10.1.3
  Delay{,3..6} (5 alike) : xbsIndex_r4/Delay  rst=off  infoeditControl=Selection of Reset will increase slice count due to use of real FFs and instead of SRLs  en=off  latency=1  dbl_ovrd=off  reg_retiming=on  block_type=delay
      settings: block_version=11.4
  Delay1 : xbsIndex_r4/Delay  rst=off  infoeditControl=Selection of Reset will increase slice count due to use of real FFs and instead of SRLs  en=off  latency=1  dbl_ovrd=off  reg_retiming=off  block_type=delay
      settings: block_version=11.4
  Delay2 : xbsIndex_r4/Delay  rst=off  infoeditControl=Selection of Reset will increase slice count due to use of real FFs and instead of SRLs  en=off  latency=1  dbl_ovrd=off  reg_retiming=off  block_type=delay
      settings: block_version=11.4
  Delay7 : xbsIndex_r4/Delay  rst=off  infoeditControl=Selection of Reset will increase slice count due to use of real FFs and instead of SRLs  en=off  latency=1  dbl_ovrd=off  reg_retiming=off  block_type=delay
      settings: block_version=11.4
  Inverter : xbsIndex_r4/Inverter  en=off  latency=1  dbl_ovrd=off  block_type=inv
      settings: block_version=11.4
  Inverter1 : xbsIndex_r4/Inverter  en=off  latency=0  dbl_ovrd=off  block_type=inv
      settings: block_version=11.4
  Logical1 : xbsIndex_r4/Logical  logical_function=AND  inputs=2  en=off  latency=1  precision=Full  arith_type=Unsigned  n_bits=8  bin_pt=2  align_bp=on  dbl_ovrd=off  block_type=logical
      settings: xl_area=[0, 0, 0, 0, 0, 0, 0]
  Logical2 : xbsIndex_r4/Logical  logical_function=AND  inputs=2  en=off  latency=1  precision=Full  arith_type=Unsigned  n_bits=8  bin_pt=2  align_bp=on  dbl_ovrd=off  block_type=logical
      settings: xl_area=[0, 0, 0, 0, 0, 0, 0]
  Logical4 : xbsIndex_r4/Logical  logical_function=OR  inputs=2  en=off  latency=0  precision=Full  arith_type=Unsigned  n_bits=8  bin_pt=2  align_bp=on  dbl_ovrd=off  block_type=logical
      settings: xl_area=[0, 0, 0, 0, 0, 0, 0]
  Logical6 : xbsIndex_r4/Logical  logical_function=AND  inputs=3  en=off  latency=0  precision=Full  arith_type=Unsigned  n_bits=8  bin_pt=2  align_bp=on  dbl_ovrd=off  block_type=logical
      settings: xl_area=[0, 0, 0, 0, 0, 0, 0]
  Register3 : xbsIndex_r4/Register  init=0  rst=on  en=on  dbl_ovrd=off  block_type=register
      settings: xl_area=[0, 0, 0, 0, 0, 0, 0]
  Register4 : xbsIndex_r4/Register  init=0  rst=off  en=on  dbl_ovrd=off  block_type=register
      settings: xl_area=[0, 0, 0, 0, 0, 0, 0]
  Register5 : xbsIndex_r4/Register  init=1  rst=on  en=on  dbl_ovrd=off  block_type=register
      settings: xl_area=[0, 0, 0, 0, 0, 0, 0]
  Shift : xbsIndex_r4/Shift  shift_dir=Left  shift_bits=32-nsamples-log2(increment)-1-1  en=off  latency=1  precision=Full  arith_type="Signed  (2's comp)"  n_bits=16  bin_pt=14  quantization=Truncate  overflow=Wrap  dbl_ovrd=off  block_type=shift
  add_gen : xbsIndex_r4/Counter  cnt_type=Free Running  cnt_to=1  operation=Up  start_count=0  cnt_by_val=increment  arith_type=Unsigned  n_bits=counter_size  bin_pt=0  load_pin=off  rst=on  en=on  explicit_period=on  period=1  dbl_ovrd=off  use_behavioral_HDL=off  implementation=DSP48  use_rpm=on  block_type=counter
      settings: xl_area=[0, 0, 0, 0, 0, 0, 0]
  edge_detect : Subsystem  AncestorBlock=casper_library_misc/edge_detect  mask(edge_detect){edge=Both, polarity=Active High, x_in=[0 0.08 0.08 0.16 0.16 0.24 0.24 0.32 0.32 0.4], y_in=[-1 -1  1  1  1  1 -1 -1 -1 -1], x_out=[0.6 0.68 0.68 0.76 0.76 0.84 0.84 0.92 0.92 1], y_out=[-1 -1  1  1 -1 -1  1  1 -1 -1]}
      mask prompts: edge "Edge Type"; polarity "Output Polarity"; x_in "Input X Positions"; y_in "Input Y Positions"; x_out "Output X Positions"; y_out "Output Y Positions"
      mask help: Output is active for one-cycle after each edge of the specified type (i.e. rising, falling or both).
      mask init: same as v01_aa_rii_16r4t11f_ver121sa/adcsnap0/add_gen/edge_detect
      settings: AttributesFormatString=|
      | both edges
      | active high
      mask Help: Output is active for one-cycle after each edge of the specified type (i.e. rising, falling, or both).  Latency is zero.
      mask StyleString: popup(Rising|Falling|Both),popup(Active High|Active Low),edit,edit,edit,edit
      mask Display:
      | plot(x_in_num,0.5+y_in_num*0.2);
      | plot(x_out_num,0.5+y_out_num*0.2);
  slice1 : xbsIndex_r4/Slice  nbits=nsamples+log2(increment)  boolean_output=off  mode=Lower Bit Location + Width  bit1=0  base1=LSB of Input  bit0=0  base0=LSB of Input  dbl_ovrd=off  block_type=slice
      settings: block_version=10.1.3
  slice2 : xbsIndex_r4/Slice  nbits=nsamples-burst_size  boolean_output=off  mode=Lower Bit Location + Width  bit1=0  base1=LSB of Input  bit0=log2(increment)+burst_size  base0=LSB of Input  dbl_ovrd=off  block_type=slice
      settings: block_version=10.1.3
  slice3 : xbsIndex_r4/Slice  nbits=1  boolean_output=on  mode=Lower Bit Location + Width  bit1=0  base1=MSB of Input  bit0=nsamples + log2(increment)  base0=LSB of Input  dbl_ovrd=off  block_type=slice
      settings: block_version=10.1.3
  vout : Outport
  add : Outport  Port=2
  dout : Outport  Port=3
  we_o : Outport  Port=4
  status : Outport  Port=5
  en_cnt : Outport  Port=6
  wires:
  Shift/1 -> Concat/1
  Delay/1 -> Register5/2
  Inverter1/1 -> Logical4/2
  cont/1 -> Delay3/1
  edge_detect/1(out) -> Inverter1/1, Register5/3
  Register4/1 -> vout/1
  Logical2/1 -> Register4/2
  Register3/1 -> Logical2/2
  vin/1 -> Delay5/1
  add_gen/1 -> slice2/1, slice1/1, Delay2/1, slice3/1
  Register5/1 -> Convert/1, Inverter/1, Logical6/1
  slice3/1 -> edge_detect/1(in)
  init/1 -> Delay4/1
  Logical6/1 -> we_o/1, Logical2/1, Register3/2, add_gen/2
  Concat/1 -> status/1
  Convert/1 -> Shift/1
  slice2/1 -> add/1
  Delay2/1 -> en_cnt/1
  din/1 -> Delay6/1
  Delay1/1 -> Concat/3
  slice1/1 -> Delay1/1
  Inverter/1 -> Concat/2
  Logical4/1 -> Logical6/2
  we/1 -> Logical1/1
  go/1 -> Logical1/2
  Logical1/1 -> Logical6/3
  Delay7/1 -> Register4/1
  Delay3/1 -> Logical4/1, Register5/1
  Delay4/1 -> Register3/3, Register3/1, add_gen/1, Delay/1
  Delay5/1 -> Delay7/1
  Delay6/1 -> dout/1
